simulink model "ofdm_txrx_mimo"  (Simulink 7.1)
settings: Solver=ode45  StartTime=0.0  StopTime=6e3  RelTol=1e-3
PreLoadFcn:
  | ofdm_rx_mimo_init
InitFcn:
  | ofdm_rx_mimo_init
StartFcn:
  | ofdm_rx_mimo_init
values from neighbouring files (symbols this model uses):
  BER_RAM_init_size = 2048    [ofdm_tx_mimo_init.m]
  CPLength = 16    [ofdm_tx_mimo_init.m]
  CRCPolynomial = hex2dec('04c11db7')    [ofdm_tx_mimo_init.m]
  freqOffset_track_filtCoef_i = hex2dec('2500')/2^32    [ofdm_rx_mimo_init.m]
  freqOffset_track_filtCoef_p = hex2dec('d0000')/2^32    [ofdm_rx_mimo_init.m]
  hi = zeros(1,length(cci))    [ofdm_rx_mimo_init.m]
  hr = zeros(1,length(ccr))    [ofdm_rx_mimo_init.m]
  max_CP_length = 16    [ofdm_tx_mimo_init.m]
  max_num_baseRateSymbols = 31    [ofdm_tx_mimo_init.m]
  max_num_subcarriers = 64    [ofdm_tx_mimo_init.m]
  max_num_trainingSymbols = 15    [ofdm_tx_mimo_init.m]
  max_numBytes = 16384    [ofdm_tx_mimo_init.m]
  max_OFDM_symbols = 2047    [ofdm_tx_mimo_init.m]
  modConstellation_qam16 = .75*[1 3 -1 -3]./3    [ofdm_tx_mimo_init.m]
  modConstellation_qam256 = 0.9375*[3 1 5 7 11 9 13 15 -3 -1 -5 -7 -11 -9 -13 -15]./15    [ofdm_tx_mimo_init.m]
  modConstellation_qam64 = 0.875*[3 1 5 7 -3 -1 -5 -7]./7    [ofdm_tx_mimo_init.m]
  modConstellation_qpsk = [1 -1]./sqrt(2)    [ofdm_tx_mimo_init.m]
  numBaseRateSymbols = 2    [ofdm_tx_mimo_init.m]
  numSubcarriers = 64    [ofdm_tx_mimo_init.m]
  numTrainingSymbols = 2    [ofdm_tx_mimo_init.m]
  pilot1_indicies = 7 + ( (64-7) * 2^16)    (= 3735559)    [ofdm_tx_mimo_init.m]
  pilot2_indicies = 21 + ( (64-21) * 2^16)    (= 2818069)    [ofdm_tx_mimo_init.m]
  pilotValue_neg = hex2dec('8000') + (2^16 * 0)    [ofdm_tx_mimo_init.m]
  pilotValue_pos = hex2dec('7FFF') + (2^16 * 0)    [ofdm_tx_mimo_init.m]
  pkt_crossCorr_thresh = 0.7    [ofdm_rx_mimo_init.m]
  pkt_energy_thresh = 0    [ofdm_rx_mimo_init.m]
  pkt_numPayloadBytes = 1400+42-6    (= 1436)    [ofdm_tx_mimo_init.m]
  pktByteNums = numHeaderBytes +  				byteIndex_numPayloadBytes(1)*2^8 +  				byteIndex_numPayloadBytes(2)*2^16 +  				byteIndex_simpleDynModMasks * 2^24    [ofdm_rx_mimo_init.m]
  PktDet_Delay = 26 + Symbol_Timing_Offset*2^7    (= 1050)    [ofdm_rx_mimo_init.m]
  preamble = 6*[0 shortsyms_10 longsyms_2]    [ofdm_tx_mimo_init.m]
  preamble_I = real(preamble)    [ofdm_tx_mimo_init.m]
  preamble_Q = imag(preamble)    [ofdm_tx_mimo_init.m]
  RAM_init_size = max_numBytes    (= 16384)    [ofdm_tx_mimo_init.m]
  reg_Interrupt_PktBuf_Control = reg_InterruptControl +  	1 * 2^16 +  	0 * 2^24    (= 65656)    [ofdm_rx_mimo_init.m]
  rx_controlBits = 1 * 2  		 + 1 * 4  		 + 0 * 8  		 + 1 * 16 * rx_SISO_Mode  		 + 1 * 32  		 + 1 * 64  		 + 0 * 128  		 + 1 * 256  		 + 0 * 512  		 + 1 * 1024  		 + 0 * 2048  		 + 1 * 4096  		 + 1 * 8192  		 + 0 * 16384  		 + 1 * 65536  		 + 1 * 131072  		 + 0 * 262144  		 + 1 * 524288    [ofdm_rx_mimo_init.m]
  Rx_PhaseNoiseTrack_K = 0.6094    [ofdm_rx_mimo_init.m]
  rx_postEq_scaling = round(rxScaling*2^11) * (1 + 2^16)    [ofdm_rx_mimo_init.m]
  RxDataScrambling_Seq = [40 198 78 63 82 173 102 245 48 111 172 115 147 230 216 93 72 65 62 2 205 242 122 90 128 83 105 97 73 10 5 252]    [ofdm_tx_mimo_init.m]
  simOnly_numSamples = length(preamble)+( (numSubcarriers+CPLength)*(numTrainingSymbols + numBaseRateSymbols + numFullRateSymbols/2) )    [ofdm_tx_mimo_init.m]
  train = [train train]    [ofdm_tx_mimo_init.m]
  tx_controlBits = (antB_preambleShift * 2^4) + (2^0 * tx_SISO_Mode) + 4*0 + 8*1    (= 73)    [ofdm_tx_mimo_init.m]
  TxDataScrambling_Seq = [40 198 78 63 82 173 102 245 48 111 172 115 147 230 216 93 72 65 62 2 205 242 122 90 128 83 105 97 73 10 5 252]    [ofdm_tx_mimo_init.m]
  txReg_symbolCounts = (2^16 * numFullRateSymbols) + (2^8 *numBaseRateSymbols ) + numTrainingSymbols    [ofdm_tx_mimo_init.m]
  TxRx_FFTScaling = bin2dec('010111') + (bin2dec('000101') * 2^6)    [ofdm_tx_mimo_init.m]
undefined symbols (not defined in the file or in its folder): AntA, AntB, BaseRate, CRC_table_gen, gcbh, intfilt, pktTiming_count_payloadStart, pktTiming_symbol_length, xlBlockLoadCallback, xlGetSimulinkPeriod
libraries: xbsIndex_r4 x4212, dspmlti4 x4, dspsigops x4, commchan3 x2, simulink x2, commrflib2 x2, dspsrcs4 x1
(part 9 of 43)

== system ofdm_txrx_mimo/OFDM Rx MIMO/Coarse Freq Correction/Coarse Freq Offset Calc Combo ==
  I In : Inport  OutDataType=sfix(16)  OutScaling=2^0
  Q In : Inport  Port=2  OutDataType=sfix(16)  OutScaling=2^0
  Reset : Inport  Port=3  OutDataType=sfix(16)  OutScaling=2^0
  PktDet : Inport  Port=4  OutDataType=sfix(16)  OutScaling=2^0
  Accum : xbsIndex_r4/Accumulator  operation=Add  n_bits=7+13  overflow=Flag as error  scale=1  rst=on  hasbypass=on  en=on  dbl_ovrd=off  use_behavioral_HDL=on  implementation=Fabric  block_type=accum
      settings: infoedit="Adder or subtractor-based accumulator.   Output type and binary point position match the input.<P><P>Hardware notes: When \"Reinitialize with input 'b' on reset\" is selected, the accumulator is forced to run at the system rate even if the input 'b' is running at a slower rate."  xl_area=[17 33 0 32 0 0 0]  block_version=VER_STRING_GOES_HERE
  Accum Control : Subsystem
  Arctan2 : Subsystem
  Coarse Freq Scope4 : Scope  NumInputPorts=5
      settings: TimeRange=3500  YMin=-5~-0.0001~0~0~0  YMax=12.5~0.00035~0.00035~1~1  SaveName=ScopeData43
  Convert1 : xbsIndex_r4/Convert  arith_type=Unsigned  n_bits=32  bin_pt=32  quantization=Truncate  overflow=Wrap  latency=0  dbl_ovrd=off  pipeline=off  block_type=convert
      settings: xl_area=[0 0 0 0 0 0 0]  block_version=VER_STRING_GOES_HERE
  Convert2 : xbsIndex_r4/Convert  arith_type="Signed  (2's comp)"  n_bits=14  bin_pt=11  quantization=Truncate  overflow=Wrap  latency=0  dbl_ovrd=off  pipeline=off  block_type=convert
      settings: xl_area=[0, 0, 0, 0, 0, 0, 0]  block_version=VER_STRING_GOES_HERE
  Convert3 : xbsIndex_r4/Convert  arith_type="Signed  (2's comp)"  n_bits=14  bin_pt=9  quantization=Truncate  overflow=Wrap  latency=0  dbl_ovrd=off  pipeline=off  block_type=convert
      settings: xl_area=[0, 0, 0, 0, 0, 0, 0]  block_version=VER_STRING_GOES_HERE
  Delay4 : xbsIndex_r4/Delay  en=off  latency=4  dbl_ovrd=off  reg_retiming=off  block_type=delay
      settings: infoedit=Hardware notes: A delay line is a chain, each link of which is an SRL16 followed by a flip-flop. If register retiming is enabled, the delay line is a chain of flip-flops.  block_version=VER_STRING_GOES_HERE
  Delay5 : xbsIndex_r4/Delay  en=off  latency=1  dbl_ovrd=off  reg_retiming=off  block_type=delay
      settings: infoedit=Hardware notes: A delay line is a chain, each link of which is an SRL16 followed by a flip-flop. If register retiming is enabled, the delay line is a chain of flip-flops.  block_version=VER_STRING_GOES_HERE
  Delay6 : xbsIndex_r4/Delay  en=off  latency=2  dbl_ovrd=off  reg_retiming=off  block_type=delay
      settings: infoedit=Hardware notes: A delay line is a chain, each link of which is an SRL16 followed by a flip-flop. If register retiming is enabled, the delay line is a chain of flip-flops.  block_version=VER_STRING_GOES_HERE
  Gateway Out{19..23} (5 alike) : xbsIndex_r4/Gateway Out  hdl_port=off  timing_constraint=None  locs_specified=off  LOCs={}  block_type=gatewayout
      settings: infoedit="Gateway out block.  Converts Xilinx fixed point inputs into ouputs of type Simulink integer, double, or fixed point.<P><P>Hardware notes:  In hardware these blocks become top level output ports or are discarded, depending on how they are configured."  xl_area=[0 0 0 0 0 0 0]  block_version=VER_STRING_GOES_HERE
  Inverter1 : xbsIndex_r4/Inverter  en=off  latency=0  dbl_ovrd=off  block_type=inv
      settings: xl_area=[0 0 0 0 0 0 0]  block_version=VER_STRING_GOES_HERE
  Logical : xbsIndex_r4/Logical  logical_function=AND  inputs=2  en=off  latency=0  precision=Full  arith_type=Unsigned  n_bits=16  bin_pt=0  align_bp=on  dbl_ovrd=off  block_type=logical
      settings: block_version=8.2.01
  Mult1 : xbsIndex_r4/Mult  precision=User Defined  arith_type="Signed  (2's comp)"  n_bits=35  bin_pt=33  quantization="Round  (unbiased: +/- Inf)"  overflow=Flag as error  en=off  latency=2  dbl_ovrd=off  use_behavioral_HDL=off  use_embedded=on  opt=Speed  optimum_pipeline=off  pipeline=off  use_rpm=off  placement_style=Rectangular shape  block_type=mult
      settings: infoedit=Hardware notes: To use the internal pipeline stage of the dedicated multiplier you must select 'Pipeline for maximum performance'.  xl_area=[52 69 0 102 0 2 0]  block_version=VER_STRING_GOES_HERE
  Mux : xbsIndex_r4/Mux  inputs=2  en=off  latency=1  precision=Full  arith_type=Unsigned  n_bits=16  bin_pt=14  quantization=Truncate  overflow=Wrap  dbl_ovrd=off  block_type=mux
      settings: block_version=8.2.01
  PhaseDiff : Subsystem
  Posedge : Subsystem
  Register4 : xbsIndex_r4/Register  init=0  rst=on  en=on  dbl_ovrd=off  block_type=register
      settings: block_version=8.2.01
  Rx_debug_CFOPhaseDiff : xbsIndex_r4/Gateway Out  hdl_port=on  timing_constraint=None  locs_specified=off  LOCs={}  block_type=gatewayout
      settings: infoedit="Gateway out block.  Converts Xilinx fixed point inputs into ouputs of type Simulink integer, double, or fixed point.<P><P>Hardware notes:  In hardware these blocks become top level output ports or are discarded, depending on how they are configured."  xl_area=[0 0 0 0 1 0 0]  block_version=VER_STRING_GOES_HERE
  Scale : xbsIndex_r4/Scale  scale_factor=-4  dbl_ovrd=off  block_type=scale
      settings: infoedit=Scales input by a power of two by adjusting the binary point position.<P><P>Hardware notes: In hardware this block costs nothing.  block_version=8.2.01
  Scale1 : xbsIndex_r4/Scale  scale_factor=-2  dbl_ovrd=off  block_type=scale
      settings: infoedit=Scales input by a power of two by adjusting the binary point position.<P><P>Hardware notes: In hardware this block costs nothing.  block_version=8.2.01
  Up Sample : xbsIndex_r4/Up Sample  sample_ratio=2  copy_samples=on  dbl_ovrd=off  block_type=usamp
      settings: infoedit="Up samples input data.  Inserted values  can be zeros or copies of the most recent input sample.<P><P>Hardware notes: No hardware is needed if inserted values are copies of the input sample; otherwise, a mux and single bit flip-flop are used."  block_version=8.2.02
  inv 2pi : xbsIndex_r4/Constant  arith_type=Unsigned  const=1/(2*pi*64*72)  n_bits=24  bin_pt=24  explicit_period=off  period=1  equ=P=C  opselect=C  inp2=PCIN>>17  opr=+  inp1=P  carry=CIN  dbl_ovrd=off  block_type=constant
      settings: block_version=VER_STRING_GOES_HERE
  Phase Inc : Outport  OutDataType=sfix(16)  OutScaling=2^0
  tag scope: CFO_PhaseDiff=global
  wires:
  PktDet/1 -> Accum Control/1(En)
  Q In/1 -> Arctan2/2(Q)
  I In/1 -> Arctan2/1(I)
  Accum Control/1(" En") -> Delay4/1
  Gateway Out19/1 -> Coarse Freq Scope4/1
  Gateway Out20/1 -> Coarse Freq Scope4/2
  Gateway Out21/1 -> Coarse Freq Scope4/3
  Gateway Out22/1 -> Coarse Freq Scope4/4
  Gateway Out23/1 -> Coarse Freq Scope4/5
  PhaseDiff/1(diff(p0,p63)) -> Accum/1, Convert3/1, [CFO_PhaseDiff]
  Inverter1/1 -> Accum/2, Posedge/1(D)
  Delay4/1 -> Delay5/1
  Accum/1 -> Mult1/1, Gateway Out22/1
  inv 2pi/1 -> Mult1/2
  Mult1/1 -> Register4/1, Gateway Out21/1
  Posedge/1(Q) -> Delay6/1
  Delay6/1 -> Gateway Out20/1, Logical/1
  Register4/1 -> Gateway Out19/1, Convert1/1
  Reset/1 -> Accum Control/2(Reset), Gateway Out23/1, Register4/2
  Arctan2/1(atan) -> PhaseDiff/1(p), Convert2/1
  Delay5/1 -> Inverter1/1, Accum/3
  Convert1/1 -> Phase Inc/1
  Logical/1 -> Register4/3
  [regRx_coarseCFO_en] -> Logical/2
  Up Sample/1 -> Rx_debug_CFOPhaseDiff/1
  Scale/1 -> Mux/3
  Mux/1 -> Up Sample/1
  [regRx_CFOdebugOutputSel] -> Mux/1
  Scale1/1 -> Mux/2
  Convert2/1 -> Scale1/1
  Convert3/1 -> Scale/1

== system ofdm_txrx_mimo/OFDM Rx MIMO/Coarse Freq Correction/DDS ==
  PhaseInc : Inport  OutDataType=sfix(16)  OutScaling=2^0
  Rst : Inport  Port=2  OutDataType=sfix(16)  OutScaling=2^0
  "DDS v4_0 " : xbsIndex_r4/DDS v4_0   Description=Direct Digital Synthesizer  mode=Sine and Cosine  neg_sin=on  neg_cos=off  n_bits=18  phase_increment_type=Register  phase_increment=1/10  phase_offset_type=Constant  phase_offset=0  rst=on  en=off  lut_addr_bits=10  acc_latency=One Cycle  acc_n_bits=32  phase_dithering=off  dbl_ovrd=off  explicit_period=off  period=1  mem_type=Block RAM  pipeline=off  use_rpm=off  block_type=ddsv4
      settings: block_version=VER_STRING_GOES_HERE
  Logical1 : xbsIndex_r4/Logical  logical_function=OR  inputs=2  en=off  latency=0  precision=Full  arith_type=Unsigned  n_bits=8  bin_pt=2  align_bp=on  dbl_ovrd=off  block_type=logical
      settings: xl_area=[1 0 0 1 0 0 0]  block_version=VER_STRING_GOES_HERE
  inv 2pi2 : xbsIndex_r4/Constant  arith_type=Boolean  const=1  n_bits=32  bin_pt=32  explicit_period=off  period=1  equ=P=C  opselect=C  inp2=PCIN>>17  opr=+  inp1=P  carry=CIN  dbl_ovrd=off  block_type=constant
      settings: block_version=VER_STRING_GOES_HERE
  Q : Outport  OutDataType=sfix(16)  OutScaling=2^0
  I : Outport  Port=2  OutDataType=sfix(16)  OutScaling=2^0
  wires:
  [regRx_freqCorrBypass] -> Logical1/2
  Logical1/1 -> "DDS v4_0 "/3
  PhaseInc/1 -> "DDS v4_0 "/1
  inv 2pi2/1 -> "DDS v4_0 "/2
  Rst/1 -> Logical1/1
  "DDS v4_0 "/1 -> Q/1
  "DDS v4_0 "/2 -> I/1

== system ofdm_txrx_mimo/OFDM Rx MIMO/Equalizer & Packetizer/CFO Loop Filter ==
  Phase Err : Inport  OutDataType=sfix(16)  OutScaling=2^0
  Rst : Inport  Port=2  OutDataType=sfix(16)  OutScaling=2^0
  Vin : Inport  Port=3  OutDataType=sfix(16)  OutScaling=2^0
  Accumulator : xbsIndex_r4/Accumulator  operation=Add  n_bits=32  overflow=Wrap  scale=1  rst=on  hasbypass=off  en=on  dbl_ovrd=off  use_behavioral_HDL=on  implementation=Fabric  block_type=accum
      settings: infoedit="Adder or subtractor-based accumulator.   Output type and binary point position match the input.<P><P>Hardware notes: When \"Reinitialize with input 'b' on reset\" is selected, the accumulator is forced to run at the system rate even if the input 'b' is running at a slower rate."  block_version=VER_STRING_GOES_HERE
  AddSub : xbsIndex_r4/AddSub  mode=Addition  use_carryin=off  use_carryout=off  en=off  latency=0  precision=User Defined  arith_type="Signed  (2's comp)"  n_bits=32  bin_pt=31  quantization=Truncate  overflow=Wrap  dbl_ovrd=off  use_behavioral_HDL=off  pipelined=off  use_rpm=on  hw_selection=Fabric  block_type=addsub
      settings: block_version=VER_STRING_GOES_HERE
  Delay : xbsIndex_r4/Delay  en=off  latency=3  dbl_ovrd=off  reg_retiming=off  block_type=delay
      settings: infoedit=Hardware notes: A delay line is a chain, each link of which is an SRL16 followed by a flip-flop. If register retiming is enabled, the delay line is a chain of flip-flops.  block_version=VER_STRING_GOES_HERE
  Display : Display
  Display1 : Display
  Down Sample1 : xbsIndex_r4/Down Sample  sample_ratio=2  sample_phase="Last Value of Frame  (most efficient)"  en=off  latency=1  dbl_ovrd=off  block_type=dsamp
      settings: block_version=VER_STRING_GOES_HERE
  Down Sample2 : xbsIndex_r4/Down Sample  sample_ratio=2  sample_phase="Last Value of Frame  (most efficient)"  en=off  latency=1  dbl_ovrd=off  block_type=dsamp
      settings: block_version=VER_STRING_GOES_HERE
  Gateway Out{1..6} (6 alike) : xbsIndex_r4/Gateway Out  hdl_port=off  timing_constraint=None  locs_specified=off  LOCs={}  block_type=gatewayout
      settings: infoedit="Gateway out block.  Converts Xilinx fixed point inputs into ouputs of type Simulink integer, double, or fixed point.<P><P>Hardware notes:  In hardware these blocks become top level output ports or are discarded, depending on how they are configured."  block_version=VER_STRING_GOES_HERE
  MIMO CFO Loop Filt : Scope  NumInputPorts=6
      settings: YMin=-3.5~-1.575e-006~-1.25e-005~-1.5e-005~0~-1  YMax=2.5~-1.375e-006~1e-005~1e-005~1~1  SaveName=ScopeData40
  Mult : xbsIndex_r4/Mult  precision=User Defined  arith_type="Signed  (2's comp)"  n_bits=32  bin_pt=31  quantization=Truncate  overflow=Wrap  en=off  latency=3  dbl_ovrd=off  use_behavioral_HDL=off  use_embedded=on  opt=Speed  optimum_pipeline=off  pipeline=on  use_rpm=off  placement_style=Rectangular shape  block_type=mult
      settings: infoedit=Hardware notes: To use the internal pipeline stage of the dedicated multiplier you must select 'Pipeline for maximum performance'.  block_version=VER_STRING_GOES_HERE
  Mult1 : xbsIndex_r4/Mult  precision=User Defined  arith_type="Signed  (2's comp)"  n_bits=32  bin_pt=31  quantization=Truncate  overflow=Wrap  en=off  latency=3  dbl_ovrd=off  use_behavioral_HDL=off  use_embedded=on  opt=Speed  optimum_pipeline=off  pipeline=on  use_rpm=off  placement_style=Rectangular shape  block_type=mult
      settings: infoedit=Hardware notes: To use the internal pipeline stage of the dedicated multiplier you must select 'Pipeline for maximum performance'.  block_version=VER_STRING_GOES_HERE
  Fine Freq : Outport  OutDataType=sfix(16)  OutScaling=2^0
  wires:
  [RxReg_FreqOffFilt_KI] -> Down Sample2/1
  Mult/1 -> Accumulator/1
  Accumulator/1 -> AddSub/1, Gateway Out1/1
  [RxReg_FreqOffFilt_KP] -> Down Sample1/1
  Phase Err/1 -> Mult1/2, Mult/1, Gateway Out2/1
  Mult1/1 -> AddSub/2, Gateway Out3/1
  AddSub/1 -> Gateway Out4/1, Fine Freq/1
  Gateway Out2/1 -> MIMO CFO Loop Filt/1
  Gateway Out1/1 -> MIMO CFO Loop Filt/2
  Gateway Out3/1 -> MIMO CFO Loop Filt/3
  Gateway Out4/1 -> MIMO CFO Loop Filt/4
  Rst/1 -> Accumulator/2, Gateway Out6/1
  Vin/1 -> Delay/1, Gateway Out5/1
  Delay/1 -> Accumulator/3
  Gateway Out5/1 -> MIMO CFO Loop Filt/5
  Gateway Out6/1 -> MIMO CFO Loop Filt/6
  Down Sample1/1 -> Mult1/1, Display/1
  Down Sample2/1 -> Mult/2, Display1/1

== system ofdm_txrx_mimo/OFDM Rx MIMO/Equalizer & Packetizer/EVM ==
  In1 : Inport  OutDataType=sfix(16)  OutScaling=2^0
  In2 : Inport  Port=2  OutDataType=sfix(16)  OutScaling=2^0
  In3 : Inport  Port=3  OutDataType=sfix(16)  OutScaling=2^0
  In4 : Inport  Orientation=down  Port=4  OutDataType=sfix(16)  OutScaling=2^0
  In5 : Inport  Orientation=down  Port=5  OutDataType=sfix(16)  OutScaling=2^0
  In6 : Inport  Orientation=down  Port=6  OutDataType=sfix(16)  OutScaling=2^0
  RST : Inport  Port=7  OutDataType=sfix(16)  OutScaling=2^0
  Accumulator : xbsIndex_r4/Accumulator  operation=Add  n_bits=32  overflow=Wrap  scale=1  rst=on  hasbypass=off  en=on  dbl_ovrd=off  use_behavioral_HDL=on  implementation=Fabric  block_type=accum
      settings: infoedit="Adder or subtractor-based accumulator.   Output type and binary point position match the input.<P><P>Hardware notes: When \"Reinitialize with input 'b' on reset\" is selected, the accumulator is forced to run at the system rate even if the input 'b' is running at a slower rate."  block_version=10.1.3
  AddSub : xbsIndex_r4/AddSub  mode=Subtraction  use_carryin=off  use_carryout=off  en=off  latency=0  precision=User Defined  arith_type="Signed  (2's comp)"  n_bits=18  bin_pt=15  quantization=Truncate  overflow=Wrap  dbl_ovrd=off  use_behavioral_HDL=off  pipelined=off  use_rpm=on  hw_selection=Fabric  block_type=addsub
      settings: block_version=10.1.3
  AddSub1 : xbsIndex_r4/AddSub  mode=Subtraction  use_carryin=off  use_carryout=off  en=off  latency=0  precision=User Defined  arith_type="Signed  (2's comp)"  n_bits=18  bin_pt=15  quantization=Truncate  overflow=Wrap  dbl_ovrd=off  use_behavioral_HDL=off  pipelined=off  use_rpm=on  hw_selection=Fabric  block_type=addsub
      settings: block_version=10.1.3
  AddSub2 : xbsIndex_r4/AddSub  mode=Addition  use_carryin=off  use_carryout=off  en=off  latency=0  precision=Full  arith_type=Unsigned  n_bits=16  bin_pt=14  quantization=Truncate  overflow=Wrap  dbl_ovrd=off  use_behavioral_HDL=off  pipelined=off  use_rpm=on  hw_selection=Fabric  block_type=addsub
      settings: block_version=10.1.3
  AddSub3 : xbsIndex_r4/AddSub  Orientation=down  mode=Subtraction  use_carryin=off  use_carryout=off  en=off  latency=0  precision=Full  arith_type=Unsigned  n_bits=16  bin_pt=14  quantization=Truncate  overflow=Wrap  dbl_ovrd=off  use_behavioral_HDL=off  pipelined=off  use_rpm=on  hw_selection=Fabric  block_type=addsub
      settings: block_version=10.1.3
  Bus Creator : BusCreator  Inputs=3
  Constant : xbsIndex_r4/Constant  arith_type=Unsigned  const=0  n_bits=4  bin_pt=0  explicit_period=off  period=1  equ=P=C  opselect=C  inp2=PCIN>>17  opr=+  inp1=P  carry=CIN  dbl_ovrd=off  block_type=constant
  Constant1 : xbsIndex_r4/Constant  Orientation=down  arith_type=Unsigned  const=1  n_bits=1  bin_pt=0  explicit_period=off  period=1  equ=P=C  opselect=C  inp2=PCIN>>17  opr=+  inp1=P  carry=CIN  dbl_ovrd=off  block_type=constant
  Constant2 : xbsIndex_r4/Constant  arith_type=Boolean  const=1  n_bits=1  bin_pt=0  explicit_period=off  period=1  equ=P=C  opselect=C  inp2=PCIN>>17  opr=+  inp1=P  carry=CIN  dbl_ovrd=off  block_type=constant
  Delay{,1..3,5} (5 alike) : xbsIndex_r4/Delay  Orientation=down  en=off  latency=2  dbl_ovrd=off  reg_retiming=off  block_type=delay
  Delay4 : xbsIndex_r4/Delay  en=off  latency=1  dbl_ovrd=off  reg_retiming=off  block_type=delay
  Delay6 : xbsIndex_r4/Delay  en=off  latency=1  dbl_ovrd=off  reg_retiming=off  block_type=delay
  Delay7 : xbsIndex_r4/Delay  Orientation=down  en=off  latency=6  dbl_ovrd=off  reg_retiming=off  block_type=delay
  Down Sample : xbsIndex_r4/Down Sample  Orientation=down  sample_ratio=80  sample_phase="Last Value of Frame  (most efficient)"  en=off  latency=1  dbl_ovrd=off  block_type=dsamp
      settings: block_version=10.1.3
  Inverter : xbsIndex_r4/Inverter  Orientation=down  en=off  latency=0  dbl_ovrd=off  block_type=inv
  Inverter1 : xbsIndex_r4/Inverter  en=off  latency=0  dbl_ovrd=off  block_type=inv
  Logical : xbsIndex_r4/Logical  logical_function=AND  inputs=2  en=off  latency=0  precision=Full  arith_type=Unsigned  n_bits=16  bin_pt=0  align_bp=on  dbl_ovrd=off  block_type=logical
  Logical1 : xbsIndex_r4/Logical  logical_function=AND  inputs=2  en=off  latency=0  precision=Full  arith_type=Unsigned  n_bits=16  bin_pt=0  align_bp=on  dbl_ovrd=off  block_type=logical
  Mult : xbsIndex_r4/Mult  precision=User Defined  arith_type="Signed  (2's comp)"  n_bits=16  bin_pt=11  quantization=Truncate  overflow=Wrap  en=off  latency=3  dbl_ovrd=off  use_behavioral_HDL=off  use_embedded=on  opt=Speed  optimum_pipeline=off  pipeline=on  use_rpm=on  placement_style=Triangular  block_type=mult
      settings: infoedit=Hardware notes: To use the internal pipeline stage of the dedicated multiplier you must select 'Pipeline for maximum performance'.  block_version=10.1.3
  Mult1 : xbsIndex_r4/Mult  precision=User Defined  arith_type="Signed  (2's comp)"  n_bits=16  bin_pt=11  quantization=Truncate  overflow=Wrap  en=off  latency=3  dbl_ovrd=off  use_behavioral_HDL=off  use_embedded=on  opt=Speed  optimum_pipeline=off  pipeline=on  use_rpm=on  placement_style=Triangular  block_type=mult
      settings: infoedit=Hardware notes: To use the internal pipeline stage of the dedicated multiplier you must select 'Pipeline for maximum performance'.  block_version=10.1.3
  Pkt Constructor Inputs1 : Scope  NumInputPorts=8
      settings: TimeRange=1800  YMin=-1.5~-1~-0.75~-0.75~0~-1~0~0  YMax=1.5~1~0.75~0.75~0.75~1~1~0.075  SaveName=ScopeData70
  Register : xbsIndex_r4/Register  init=0  rst=on  en=off  dbl_ovrd=off  block_type=register
  Register1 : xbsIndex_r4/Register  init=0  rst=off  en=on  dbl_ovrd=off  block_type=register
  Register2 : xbsIndex_r4/Register  init=0  rst=on  en=on  dbl_ovrd=off  block_type=register
  Relational : xbsIndex_r4/Relational  mode=a!=b  en=off  latency=1  dbl_ovrd=off  block_type=relational
      settings: block_version=10.1.3
  Relational1 : xbsIndex_r4/Relational  mode=a>b  en=off  latency=1  dbl_ovrd=off  block_type=relational
      settings: block_version=10.1.3
  To Register : xbsIndex_r4/To Register  shared_memory_name='Rx_collision'  init=0  ownership=Locally owned and initialized  explicit_data_type=on  arith_type=Unsigned  n_bits=1  bin_pt=0  dbl_ovrd=off  block_type=toreg
      settings: AttributesFormatString=<< %<shared_memory_name> >>  infoedit="Register block that writes data to a shared memory register.  Delay of one sample period."  block_version=10.1.3
  To Workspace : ToWorkspace  VariableName=simout  SampleTime=-1  SaveFormat=Structure
  done{2..5,11} (5 alike) : xbsIndex_r4/Gateway Out  hdl_port=off  timing_constraint=None  locs_specified=off  LOCs={}  block_type=gatewayout
      settings: infoedit="Gateway out block.  Converts Xilinx fixed point inputs into ouputs of type Simulink integer, double, or fixed point.<P><P>Hardware notes:  In hardware these blocks become top level output ports or are discarded, depending on how they are configured."  xl_area=[0 0 0 0 1 0 0]  block_version=VER_STRING_GOES_HERE
  wires:
  In1/1 -> done4/1, AddSub/2
  In2/1 -> done5/1, AddSub1/2
  AddSub/1 -> Mult/1, Mult/2
  AddSub1/1 -> Mult1/1, Mult1/2
  Mult/1 -> AddSub2/1
  Mult1/1 -> AddSub2/2
  AddSub2/1 -> Register/1
  Delay/1 -> AddSub/1, done2/1
  Delay1/1 -> AddSub1/1, done3/1
  Delay2/1 -> Delay4/1
  Constant/1 -> Relational/2
  Relational/1 -> Logical/1
  Logical/1 -> Delay3/1
  done2/1 -> Pkt Constructor Inputs1/1
  done3/1 -> Pkt Constructor Inputs1/2
  done4/1 -> Pkt Constructor Inputs1/3
  done5/1 -> Pkt Constructor Inputs1/4
  done11/1 -> Pkt Constructor Inputs1/5
  Register/1 -> Pkt Constructor Inputs1/8, Accumulator/1, Bus Creator/3
  Delay4/1 -> Logical/2, Delay5/1
  Delay3/1 -> Inverter/1, Accumulator/3
  Inverter/1 -> Register/2, Pkt Constructor Inputs1/7
  In4/1 -> Delay/1
  In5/1 -> Delay1/1
  In6/1 -> Delay2/1
  In3/1 -> Relational/1
  Delay5/1 -> Inverter1/1, Delay6/1
  Inverter1/1 -> Accumulator/2, Logical1/1
  Accumulator/1 -> Register1/1
  Register1/1 -> Down Sample/1, Bus Creator/2
  Delay6/1 -> Logical1/2
  Logical1/1 -> Register1/2
  Down Sample/1 -> AddSub3/1, Delay7/1
  Delay7/1 -> AddSub3/2
  AddSub3/1 -> done11/1, Bus Creator/1, Relational1/1
  Bus Creator/1 -> To Workspace/1
  [RxReg_EVM] -> Relational1/2
  Relational1/1 -> Register2/3
  Constant1/1 -> Register2/1
  RST/1 -> Register2/2
  Constant2/1 -> To Register/2
  Register2/1 -> To Register/1
  To Register/1 -> Pkt Constructor Inputs1/6

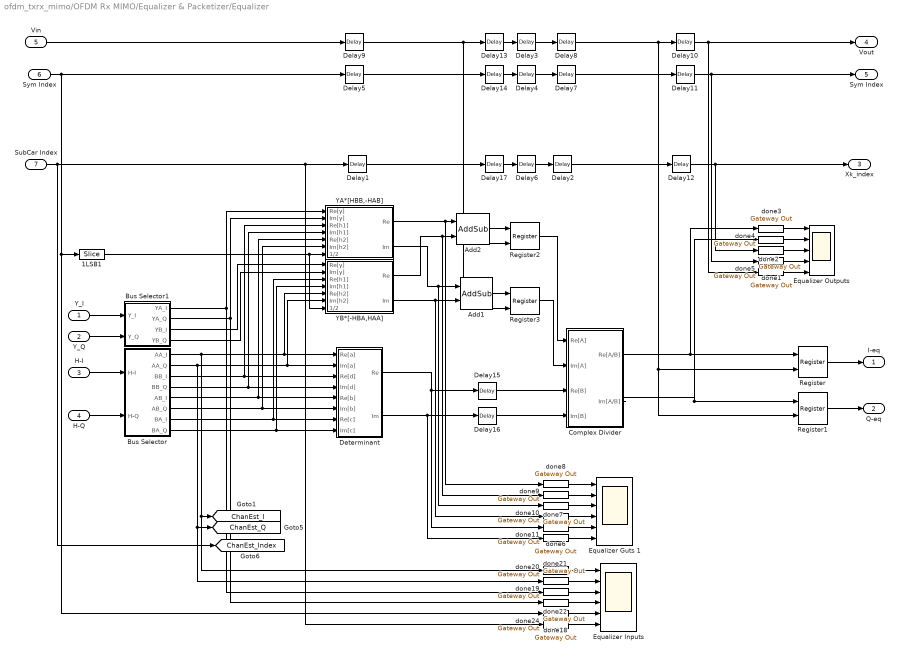
== system ofdm_txrx_mimo/OFDM Rx MIMO/Equalizer & Packetizer/Equalizer ==
  Y_I : Inport  OutDataType=sfix(16)  OutScaling=2^0
  Y_Q : Inport  Port=2  OutDataType=sfix(16)  OutScaling=2^0
  H-I : Inport  Port=3  OutDataType=sfix(16)  OutScaling=2^0
  H-Q : Inport  Port=4  OutDataType=sfix(16)  OutScaling=2^0
  Vin : Inport  Port=5  OutDataType=sfix(16)  OutScaling=2^0
  Sym Index : Inport  Port=6  OutDataType=sfix(16)  OutScaling=2^0
  SubCar Index : Inport  Port=7  OutDataType=sfix(16)  OutScaling=2^0
  1LSB1 : xbsIndex_r4/Slice  nbits=1  boolean_output=off  mode=Lower Bit Location + Width  bit1=0  base1=MSB of Input  bit0=0  base0=LSB of Input  dbl_ovrd=off  block_type=slice
      settings: infoedit="Extracts a given range of bits from each input sample and presents it at the output.  The output type is ordinarily unsigned with binary point at zero, but can be Boolean when the slice is one bit wide.<P><P>Hardware notes: In hardware this block costs nothing."  block_version=VER_STRING_GOES_HERE
  Add1 : xbsIndex_r4/AddSub  mode=Addition  use_carryin=off  use_carryout=off  en=off  latency=0  precision=Full  arith_type="Signed  (2's comp)"  n_bits=18  bin_pt=16  quantization=Truncate  overflow=Flag as error  dbl_ovrd=off  use_behavioral_HDL=on  pipelined=off  use_rpm=on  hw_selection=Fabric  block_type=addsub
      settings: xl_area=[9 0 0 16 0 0 0]  block_version=VER_STRING_GOES_HERE
  Add2 : xbsIndex_r4/AddSub  mode=Addition  use_carryin=off  use_carryout=off  en=off  latency=0  precision=Full  arith_type="Signed  (2's comp)"  n_bits=18  bin_pt=16  quantization=Truncate  overflow=Flag as error  dbl_ovrd=off  use_behavioral_HDL=on  pipelined=off  use_rpm=on  hw_selection=Fabric  block_type=addsub
      settings: xl_area=[9 0 0 16 0 0 0]  block_version=VER_STRING_GOES_HERE
  Bus Selector : Subsystem
  Bus Selector1 : Subsystem
  Complex Divider : Subsystem
  Delay{1,3..6,9} (6 alike) : xbsIndex_r4/Delay  en=off  latency=2  dbl_ovrd=off  reg_retiming=off  block_type=delay
      settings: infoedit=Hardware notes: A delay line is a chain, each link of which is an SRL16 followed by a flip-flop. If register retiming is enabled, the delay line is a chain of flip-flops.  xl_area=[0, 0, 0, 0, 0, 0, 0]  block_version=VER_STRING_GOES_HERE
  Delay{10..17} (8 alike) : xbsIndex_r4/Delay  en=off  latency=1  dbl_ovrd=off  reg_retiming=off  block_type=delay
      settings: infoedit=Hardware notes: A delay line is a chain, each link of which is an SRL16 followed by a flip-flop. If register retiming is enabled, the delay line is a chain of flip-flops.  xl_area=[0, 0, 0, 0, 0, 0, 0]  block_version=VER_STRING_GOES_HERE
  Delay2 : xbsIndex_r4/Delay  en=off  latency=19+2  dbl_ovrd=off  reg_retiming=off  block_type=delay
      settings: infoedit=Hardware notes: A delay line is a chain, each link of which is an SRL16 followed by a flip-flop. If register retiming is enabled, the delay line is a chain of flip-flops.  xl_area=[0, 0, 0, 0, 0, 0, 0]  block_version=VER_STRING_GOES_HERE
  Delay7 : xbsIndex_r4/Delay  en=off  latency=19+2  dbl_ovrd=off  reg_retiming=off  block_type=delay
      settings: infoedit=Hardware notes: A delay line is a chain, each link of which is an SRL16 followed by a flip-flop. If register retiming is enabled, the delay line is a chain of flip-flops.  xl_area=[0, 0, 0, 0, 0, 0, 0]  block_version=VER_STRING_GOES_HERE
  Delay8 : xbsIndex_r4/Delay  en=off  latency=19+2  dbl_ovrd=off  reg_retiming=off  block_type=delay
      settings: infoedit=Hardware notes: A delay line is a chain, each link of which is an SRL16 followed by a flip-flop. If register retiming is enabled, the delay line is a chain of flip-flops.  xl_area=[0, 0, 0, 0, 0, 0, 0]  block_version=VER_STRING_GOES_HERE
  Determinant : Subsystem
  Equalizer Outputs : Scope  NumInputPorts=5
      settings: TimeRange=4000  YMin=-0.0008~-0.0007~10~22.8~0.95  YMax=0.0007~0.0007~60~25.2~1.05  SaveName=ScopeData26
  Equalizer Guts 1 : Scope  NumInputPorts=6
      settings: TimeRange=5000  YMin=0~-0.001~-0.4~-0.2~0~0  YMax=0.8~0.001~0.4~0.8~1~80  SaveName=ScopeData58
  Equalizer Inputs : Scope  NumInputPorts=6
      settings: TimeRange=5000  YMin=0~-0.001~-0.4~-0.2~0~0  YMax=0.8~0.001~0.4~0.8~1~80  SaveName=ScopeData18
  Register{,1..3} (4 alike) : xbsIndex_r4/Register  init=0  rst=off  en=on  dbl_ovrd=off  block_type=register
      Register settings: block_version=VER_STRING_GOES_HERE
      Register1 settings: block_version=VER_STRING_GOES_HERE
      Register2 settings: block_version=8.2.02
      Register3 settings: block_version=8.2.02
  YA*[HBB,-HAB] : Subsystem
  YB*[-HBA,HAA] : Subsystem
  done{1..11,18..22,24} (17 alike) : xbsIndex_r4/Gateway Out  hdl_port=off  timing_constraint=None  locs_specified=off  LOCs={}  block_type=gatewayout
      settings: infoedit="Gateway out block.  Converts Xilinx fixed point inputs into ouputs of type Simulink integer, double, or fixed point.<P><P>Hardware notes:  In hardware these blocks become top level output ports or are discarded, depending on how they are configured."  xl_area=[0 0 0 0 1 0 0]  block_version=VER_STRING_GOES_HERE
  I-eq : Outport  OutDataType=sfix(16)  OutScaling=2^0
  Q-eq : Outport  Port=2  OutDataType=sfix(16)  OutScaling=2^0
  " Xk_index" : Outport  Port=3  OutDataType=sfix(16)  OutScaling=2^0
  Vout : Outport  Port=4  OutDataType=sfix(16)  OutScaling=2^0
  " Sym Index" : Outport  Port=5  OutDataType=sfix(16)  OutScaling=2^0
  tag scope: ChanEst_I=global, ChanEst_Index=global, ChanEst_Q=global
  wires:
  H-I/1 -> Bus Selector/1(H-I)
  H-Q/1 -> Bus Selector/2(H-Q)
  SubCar Index/1 -> [ChanEst_Index], Delay1/1, done24/1
  Delay6/1 -> Delay2/1
  done18/1 -> Equalizer Inputs/5
  done20/1 -> Equalizer Inputs/1
  done21/1 -> Equalizer Inputs/2
  done19/1 -> Equalizer Inputs/3
  done22/1 -> Equalizer Inputs/4
  done24/1 -> Equalizer Inputs/6
  done3/1 -> Equalizer Outputs/1
  done4/1 -> Equalizer Outputs/2
  done2/1 -> Equalizer Outputs/3
  Vin/1 -> Delay9/1
  Delay3/1 -> Delay8/1
  Delay4/1 -> Delay7/1
  done1/1 -> Equalizer Outputs/4
  Delay2/1 -> Delay12/1
  Delay7/1 -> Delay11/1
  Delay8/1 -> Register1/2, Register/2, Delay10/1
  Sym Index/1 -> Delay5/1, 1LSB1/1, done18/1
  Bus Selector/1(AA_I) -> Determinant/1(Re[a]), YB*[-HBA,HAA]/5(Re[h2]), done20/1, [ChanEst_I]
  Bus Selector/2(AA_Q) -> Determinant/2(Im[a]), YB*[-HBA,HAA]/6(Im[h2]), done21/1, [ChanEst_Q]
  Bus Selector/3(BB_I) -> Determinant/3(Re[d]), YA*[HBB,-HAB]/3(Re[h1])
  Bus Selector/4(BB_Q) -> Determinant/4(Im[d]), YA*[HBB,-HAB]/4(Im[h1])
  Bus Selector/5(AB_I) -> Determinant/5(Re[b]), YA*[HBB,-HAB]/5(Re[h2])
  Bus Selector/6(AB_Q) -> Determinant/6(Im[b]), YA*[HBB,-HAB]/6(Im[h2])
  Bus Selector/7(BA_I) -> Determinant/7(Re[c]), YB*[-HBA,HAA]/3(Re[h1])
  Bus Selector/8(BA_Q) -> Determinant/8(Im[c]), YB*[-HBA,HAA]/4(Im[h1])
  Determinant/1(Re) -> done6/1, Delay15/1
  Determinant/2(Im) -> done11/1, Delay16/1
  Y_I/1 -> Bus Selector1/1(Y_I)
  Y_Q/1 -> Bus Selector1/2(Y_Q)
  Bus Selector1/1(YA_I) -> YA*[HBB,-HAB]/1(Re[y]), done19/1
  Bus Selector1/2(YA_Q) -> YA*[HBB,-HAB]/2(Im[y]), done22/1
  Bus Selector1/3(YB_I) -> YB*[-HBA,HAA]/1(Re[y])
  Bus Selector1/4(YB_Q) -> YB*[-HBA,HAA]/2(Im[y])
  YA*[HBB,-HAB]/1(Re) -> done8/1, Add2/1
  YB*[-HBA,HAA]/1(Re) -> done9/1, Add2/2
  YA*[HBB,-HAB]/2(Im) -> done7/1, Add1/1
  YB*[-HBA,HAA]/2(Im) -> done10/1, Add1/2
  Delay1/1 -> Delay17/1
  Delay5/1 -> Delay14/1
  Delay9/1 -> Delay13/1, Register2/2, Register3/2
  done5/1 -> Equalizer Outputs/5
  Complex Divider/1(Re[A/B]) -> Register/1, done3/1
  Register/1 -> I-eq/1
  Complex Divider/2(Im[A/B]) -> Register1/1, done4/1
  Register1/1 -> Q-eq/1
  Delay10/1 -> Vout/1, done5/1
  Delay11/1 -> " Sym Index"/1, done1/1
  Delay12/1 -> " Xk_index"/1, done2/1
  done6/1 -> Equalizer Guts 1/5
  done8/1 -> Equalizer Guts 1/1
  done9/1 -> Equalizer Guts 1/2
  done7/1 -> Equalizer Guts 1/3
  done10/1 -> Equalizer Guts 1/4
  done11/1 -> Equalizer Guts 1/6
  1LSB1/1 -> YA*[HBB,-HAB]/7(1/2), YB*[-HBA,HAA]/7(1/2)
  Add2/1 -> Register2/1
  Add1/1 -> Register3/1
  Delay14/1 -> Delay4/1
  Delay13/1 -> Delay3/1
  Register2/1 -> Complex Divider/1(Re[A])
  Register3/1 -> Complex Divider/2(Im[A])
  Delay15/1 -> Complex Divider/3(Re[B])
  Delay16/1 -> Complex Divider/4(Im[B])
  Delay17/1 -> Delay6/1

== system ofdm_txrx_mimo/OFDM Rx MIMO/Equalizer & Packetizer/Packet_Constructor ==
  note: The index keeps track of the first unused location in the buffer, indexed from zero.
      |   I.e. if there are 5 bits in the buffer occupying 0 to 4, the index will be 5.
      | This is used to select which byte out of the 15 bits in the buffer will make it into the RAM block.
  I : Inport  OutDataType=sfix(16)  OutScaling=2^0
  Q : Inport  Port=2  OutDataType=sfix(16)  OutScaling=2^0
  Xk_index : Inport  Port=3  OutDataType=sfix(16)  OutScaling=2^0
  Vin : Inport  Port=4  OutDataType=sfix(16)  OutScaling=2^0
  Sym Index : Inport  Port=5  OutDataType=sfix(16)  OutScaling=2^0
  Start : Inport  Port=6  OutDataType=sfix(16)  OutScaling=2^0
  "  " : Subsystem  mask{period=1}
  >=8? : xbsIndex_r4/Slice  Orientation=up  nbits=1  boolean_output=off  mode=Lower Bit Location + Width  bit1=0  base1=MSB of Input  bit0=3  base0=LSB of Input  dbl_ovrd=off  block_type=slice
      settings: infoedit="Extracts a given range of bits from each input sample and presents it at the output.  The output type is ordinarily unsigned with binary point at zero, but can be Boolean when the slice is one bit wide.<P><P>Hardware notes: In hardware this block costs nothing."  block_version=VER_STRING_GOES_HERE
  AddSub1 : xbsIndex_r4/AddSub  mode=Subtraction  use_carryin=off  use_carryout=off  en=off  latency=0  precision=User Defined  arith_type=Unsigned  n_bits=4  bin_pt=0  quantization=Truncate  overflow=Wrap  dbl_ovrd=off  use_behavioral_HDL=on  pipelined=off  use_rpm=on  hw_selection=Fabric  block_type=addsub
      settings: xl_area=[2 0 0 4 0 0 0]  block_version=VER_STRING_GOES_HERE
  AddSub3 : xbsIndex_r4/AddSub  mode=Addition  use_carryin=off  use_carryout=off  en=off  latency=0  precision=User Defined  arith_type=Unsigned  n_bits=4  bin_pt=0  quantization=Truncate  overflow=Wrap  dbl_ovrd=off  use_behavioral_HDL=on  pipelined=off  use_rpm=on  hw_selection=Fabric  block_type=addsub
      settings: xl_area=[2 0 0 4 0 0 0]  block_version=VER_STRING_GOES_HERE
  Assert1 : xbsIndex_r4/Assert  Orientation=left  assert_type=off  type_source=Explicitly  arith_type="Signed  (2's comp)"  n_bits=16  bin_pt=14  assert_rate=on  rate_source=Explicitly  period=2  output_port=on  block_type=assert
      settings: infoedit=Asserts a user-defined sample rate and/or type on a signal.<P><P>Hardware notes: In hardware this block costs nothing.  block_version=VER_STRING_GOES_HERE
  Assert2 : xbsIndex_r4/Assert  assert_type=off  type_source=Explicitly  arith_type="Signed  (2's comp)"  n_bits=16  bin_pt=14  assert_rate=on  rate_source=Explicitly  period=2  output_port=on  block_type=assert
      settings: infoedit=Asserts a user-defined sample rate and/or type on a signal.<P><P>Hardware notes: In hardware this block costs nothing.  block_version=VER_STRING_GOES_HERE
  Barrel Shifter : Subsystem
  Convert2 : xbsIndex_r4/Convert  Orientation=down  arith_type=Boolean  n_bits=8  bin_pt=6  quantization=Truncate  overflow=Wrap  latency=0  dbl_ovrd=off  pipeline=off  block_type=convert
      settings: xl_area=[0 0 0 0 0 0 0]  block_version=VER_STRING_GOES_HERE
  Convert3 : xbsIndex_r4/Convert  Orientation=down  arith_type=Unsigned  n_bits=3  bin_pt=0  quantization=Truncate  overflow=Wrap  latency=0  dbl_ovrd=off  pipeline=off  block_type=convert
      settings: xl_area=[0 0 0 0 0 0 0]  block_version=VER_STRING_GOES_HERE
  Counter1 : xbsIndex_r4/Counter  cnt_type=Free Running  cnt_to=Inf  operation=Up  start_count=0  cnt_by_val=1  arith_type=Unsigned  n_bits=ceil(log2(RAM_init_size))  bin_pt=0  load_pin=off  rst=on  en=on  explicit_period=off  period=2  dbl_ovrd=off  use_behavioral_HDL=off  use_rpm=on  implementation=Fabric  block_type=counter
      settings: xl_area=[5 10 0 10 0 0 0]  block_version=VER_STRING_GOES_HERE
  Data Buffer : Subsystem
  Delay1 : xbsIndex_r4/Delay  en=off  latency=1  dbl_ovrd=off  reg_retiming=off  block_type=delay
      settings: infoedit=Hardware notes: A delay line is a chain, each link of which is an SRL16 followed by a flip-flop. If register retiming is enabled, the delay line is a chain of flip-flops.  xl_area=[1 1 0 0 0 0 0]  block_version=VER_STRING_GOES_HERE
  Delay2 : xbsIndex_r4/Delay  en=off  latency=1  dbl_ovrd=off  reg_retiming=off  block_type=delay
      settings: infoedit=Hardware notes: A delay line is a chain, each link of which is an SRL16 followed by a flip-flop. If register retiming is enabled, the delay line is a chain of flip-flops.  xl_area=[1 1 0 0 0 0 0]  block_version=VER_STRING_GOES_HERE
  Delay3 : xbsIndex_r4/Delay  en=off  latency=1  dbl_ovrd=off  reg_retiming=off  block_type=delay
      settings: infoedit=Hardware notes: A delay line is a chain, each link of which is an SRL16 followed by a flip-flop. If register retiming is enabled, the delay line is a chain of flip-flops.  xl_area=[1 1 0 0 0 0 0]  block_version=VER_STRING_GOES_HERE
  Hard Decision : Subsystem
  Inverter : xbsIndex_r4/Inverter  en=off  latency=0  dbl_ovrd=off  block_type=inv
      settings: xl_area=[0 0 0 0 0 0 0]  block_version=VER_STRING_GOES_HERE
  Inverter1 : xbsIndex_r4/Inverter  en=off  latency=0  dbl_ovrd=off  block_type=inv
      settings: xl_area=[0 0 0 0 0 0 0]  block_version=VER_STRING_GOES_HERE
  Logical1 : xbsIndex_r4/Logical  Orientation=down  logical_function=AND  inputs=3  en=off  latency=0  precision=Full  arith_type=Unsigned  n_bits=8  bin_pt=2  align_bp=on  dbl_ovrd=off  block_type=logical
      settings: xl_area=[1 0 0 1 0 0 0]  block_version=VER_STRING_GOES_HERE
  Logical2 : xbsIndex_r4/Logical  logical_function=AND  inputs=3  en=off  latency=0  precision=Full  arith_type=Unsigned  n_bits=8  bin_pt=2  align_bp=on  dbl_ovrd=off  block_type=logical
      settings: xl_area=[1 0 0 1 0 0 0]  block_version=VER_STRING_GOES_HERE
  Logical3 : xbsIndex_r4/Logical  logical_function=AND  inputs=2  en=off  latency=0  precision=Full  arith_type=Unsigned  n_bits=8  bin_pt=2  align_bp=on  dbl_ovrd=off  block_type=logical
      settings: xl_area=[1 0 0 1 0 0 0]  block_version=VER_STRING_GOES_HERE
  Logical4 : xbsIndex_r4/Logical  logical_function=OR  inputs=3  en=off  latency=0  precision=Full  arith_type=Unsigned  n_bits=8  bin_pt=2  align_bp=on  dbl_ovrd=off  block_type=logical
      settings: xl_area=[4 0 0 4 0 0 0]  block_version=VER_STRING_GOES_HERE
  Modulation RAM : Subsystem
  Pkt Interrupt Outputs : Subsystem
  Register : xbsIndex_r4/Register  init=0  rst=off  en=off  dbl_ovrd=off  block_type=register
      settings: block_version=8.2.02
  Relational : xbsIndex_r4/Relational  mode=a<b  en=off  latency=0  dbl_ovrd=off  block_type=relational
      settings: xl_area=[6 0 0 11 0 0 0]  block_version=VER_STRING_GOES_HERE
  Shift8 : xbsIndex_r4/Shift  Orientation=left  shift_dir=Left  shift_bits=3  en=off  latency=0  precision=User Defined  arith_type=Unsigned  n_bits=4  bin_pt=0  quantization=Truncate  overflow=Wrap  dbl_ovrd=off  block_type=shift
      settings: xl_area=[0 0 0 0 0 0 0]  block_version=VER_STRING_GOES_HERE
  Slicer : Subsystem
  index acc1 : xbsIndex_r4/Accumulator  operation=Add  n_bits=4  overflow=Wrap  scale=1  rst=on  hasbypass=off  en=on  dbl_ovrd=off  use_behavioral_HDL=on  implementation=Fabric  block_type=accum
      settings: infoedit="Adder or subtractor-based accumulator.   Output type and binary point position match the input.<P><P>Hardware notes: When \"Reinitialize with input 'b' on reset\" is selected, the accumulator is forced to run at the system rate even if the input 'b' is running at a slower rate."  xl_area=[2 4 0 4 0 0 0]  block_version=VER_STRING_GOES_HERE
  done : Outport  OutDataType=sfix(16)  OutScaling=2^0
  IOUT : Outport  Port=2  OutDataType=sfix(16)  OutScaling=2^0
  QOUT : Outport  Port=3  OutDataType=sfix(16)  OutScaling=2^0
  MOD : Outport  Port=4  OutDataType=sfix(16)  OutScaling=2^0
  tag scope: EQ_I=global, EQ_Q=global
  wires:
  Delay2/1 -> AddSub1/1, AddSub3/1, Barrel Shifter/1(ShiftSel)
  Data Buffer/1(numBytes) -> Relational/2
  Logical4/1 -> index acc1/2, Counter1/1, Data Buffer/4(Rst)
  AddSub1/1 -> index acc1/1
  Shift8/1 -> AddSub1/2
  index acc1/1 -> AddSub3/2
  AddSub3/1 -> Assert2/1
  >=8?/1 -> Shift8/1, Convert2/1
  Barrel Shifter/1(Q) -> Slicer/2(D)
  Logical2/1 -> Data Buffer/2(Payload_WE)
  Slicer/1(Q) -> Data Buffer/3(Payload_Data)
  "  "/1(pulse) -> Register/1
  Inverter/1 -> "  "/1(signal)
  Convert2/1 -> Logical1/2, Logical2/2
  Logical1/1 -> Counter1/2
  Logical3/1 -> Logical2/1, Logical1/3, Inverter/1
  Counter1/1 -> Data Buffer/1(Payload_Addr), Relational/1
  Convert3/1 -> Slicer/1(SliceSel)
  Modulation RAM/1(mod) -> Hard Decision/1(Mod Sel), Delay2/1
  Xk_index/1 -> Modulation RAM/1(Xk_index)
  Sym Index/1 -> Modulation RAM/2(Sym Index)
  Hard Decision/1(bits) -> Barrel Shifter/2(D)
  I/1 -> Hard Decision/2(I), [EQ_I]
  Q/1 -> Hard Decision/3(Q), [EQ_Q]
  Start/1 -> Logical4/1
  [rx_global_reset] -> Logical4/2, Logical4/3
  Data Buffer/2(CRCError) -> Pkt Interrupt Outputs/2(CRC-Err)
  Assert2/1 -> Convert3/1, >=8?/1
  Vin/1 -> Delay3/1
  Delay1/1 -> Barrel Shifter/3(en), index acc1/3, Logical1/1, Logical2/3
  Delay3/1 -> Delay1/1
  Register/1 -> done/1, Pkt Interrupt Outputs/1(PktDone)
  Data Buffer/6(modMask) -> Modulation RAM/3(FullRateMasks)
  Data Buffer/5(ResetRx) -> Assert1/1
  Relational/1 -> Logical3/1
  Inverter1/1 -> Logical3/2
  Assert1/1 -> Inverter1/1
  Data Buffer/3(GoodHeader) -> Pkt Interrupt Outputs/3(GoodHeader)
  Data Buffer/4(BadHeader) -> Pkt Interrupt Outputs/4(BadHeader)
  Hard Decision/2(IOUT) -> IOUT/1
  Hard Decision/3(QOUT) -> QOUT/1
  Hard Decision/4(MOD) -> MOD/1

== system ofdm_txrx_mimo/OFDM Rx MIMO/Equalizer & Packetizer/Phase Adjust ==
  Phase : Inport  OutDataType=sfix(16)  OutScaling=2^0
  I : Inport  Port=2  OutDataType=sfix(16)  OutScaling=2^0
  Q : Inport  Port=3  OutDataType=sfix(16)  OutScaling=2^0
  SubCar Index : Inport  Port=4  OutDataType=sfix(16)  OutScaling=2^0
  Vin : Inport  Port=5  OutDataType=sfix(16)  OutScaling=2^0
  Sym Index : Inport  Port=6  OutDataType=sfix(16)  OutScaling=2^0
  ComplexMult_x1 : Subsystem
  Delay{1..4} (4 alike) : xbsIndex_r4/Delay  en=off  latency=1  dbl_ovrd=off  reg_retiming=off  block_type=delay
      settings: infoedit=Hardware notes: A delay line is a chain, each link of which is an SRL16 followed by a flip-flop. If register retiming is enabled, the delay line is a chain of flip-flops.  xl_area=[0, 0, 0, 0, 0, 0, 0]  block_version=VER_STRING_GOES_HERE
  Delay5 : xbsIndex_r4/Delay  en=off  latency=4  dbl_ovrd=off  reg_retiming=off  block_type=delay
      settings: infoedit=Hardware notes: A delay line is a chain, each link of which is an SRL16 followed by a flip-flop. If register retiming is enabled, the delay line is a chain of flip-flops.  xl_area=[0, 0, 0, 0, 0, 0, 0]  block_version=VER_STRING_GOES_HERE
  Delay6 : xbsIndex_r4/Delay  en=off  latency=4  dbl_ovrd=off  reg_retiming=off  block_type=delay
      settings: infoedit=Hardware notes: A delay line is a chain, each link of which is an SRL16 followed by a flip-flop. If register retiming is enabled, the delay line is a chain of flip-flops.  xl_area=[0, 0, 0, 0, 0, 0, 0]  block_version=VER_STRING_GOES_HERE
  Delay7 : xbsIndex_r4/Delay  en=off  latency=4  dbl_ovrd=off  reg_retiming=off  block_type=delay
      settings: infoedit=Hardware notes: A delay line is a chain, each link of which is an SRL16 followed by a flip-flop. If register retiming is enabled, the delay line is a chain of flip-flops.  xl_area=[0, 0, 0, 0, 0, 0, 0]  block_version=VER_STRING_GOES_HERE
  Phase Adjust : Scope  NumInputPorts=7
      settings: TimeRange=1800  YMin=-0.75~0.425~-1~-1.25~-1.25~-1.25~-1  YMax=0.75~0.65~1~1.25~1.25~1.25~1  SaveName=ScopeData67
  Phase Calc : Subsystem
  SineCosine : xbsIndex_r4/SineCosine  mode=Sine and Cosine  neg_sin=on  neg_cos=off  n_bits=16  sym_output=off  en=off  latency=1  dbl_ovrd=off  explicit_period=off  period=1  mem_type=Block RAM  pipeline=off  use_rpm=on  block_type=sincos
      settings: block_version=8.2.02
  done{1..2,6..10} (7 alike) : xbsIndex_r4/Gateway Out  hdl_port=off  timing_constraint=None  locs_specified=off  LOCs={}  block_type=gatewayout
      settings: infoedit="Gateway out block.  Converts Xilinx fixed point inputs into ouputs of type Simulink integer, double, or fixed point.<P><P>Hardware notes:  In hardware these blocks become top level output ports or are discarded, depending on how they are configured."  xl_area=[0 0 0 0 1 0 0]  block_version=VER_STRING_GOES_HERE
  " I" : Outport  OutDataType=sfix(16)  OutScaling=2^0
  " Q" : Outport  Port=2  OutDataType=sfix(16)  OutScaling=2^0
  " SubCar Index" : Outport  Port=3  OutDataType=sfix(16)  OutScaling=2^0
  Vout : Outport  Port=4  OutDataType=sfix(16)  OutScaling=2^0
  " Sym Index" : Outport  Port=5  OutDataType=sfix(16)  OutScaling=2^0
  wires:
  SineCosine/2 -> done7/1, ComplexMult_x1/3(Re[B])
  Vin/1 -> Delay5/1
  Sym Index/1 -> Delay6/1
  SubCar Index/1 -> Delay7/1
  Delay7/1 -> " SubCar Index"/1
  Delay6/1 -> " Sym Index"/1
  Delay5/1 -> Vout/1
  Delay1/1 -> ComplexMult_x1/1(Re[A]), done8/1
  Delay2/1 -> ComplexMult_x1/2(Im[A]), done9/1
  SineCosine/1 -> ComplexMult_x1/4(Im[B]), done1/1
  done6/1 -> Phase Adjust/1
  done7/1 -> Phase Adjust/2
  done1/1 -> Phase Adjust/3
  done8/1 -> Phase Adjust/4
  done9/1 -> Phase Adjust/5
  done10/1 -> Phase Adjust/6
  done2/1 -> Phase Adjust/7
  ComplexMult_x1/1(Re) -> done10/1, " I"/1
  ComplexMult_x1/2(Im) -> done2/1, " Q"/1
  I/1 -> Delay4/1
  Q/1 -> Delay3/1
  Phase Calc/1(" Phase") -> SineCosine/1, done6/1
  Phase/1 -> Phase Calc/1(Phase/k)
  Delay3/1 -> Delay2/1
  Delay4/1 -> Delay1/1

== system ofdm_txrx_mimo/OFDM Rx MIMO/Equalizer & Packetizer/Phase Noise Tracker ==
  note: http://ieeexplore.ieee.org/xpls/abs_all.jsp?arnumber=1683187
  note: Adapted from the phase tracking system described by Akita, Sakata and Sato
  note: http://sciencelinks.jp/j-east/article/200415/000020041504A0494348.php
  note: http://ieeexplore.ieee.org/xpls/abs_all.jsp?arnumber=1405003
  Phase Err : Inport  OutDataType=sfix(16)  OutScaling=2^0
  Reset : Inport  Port=2  OutDataType=sfix(16)  OutScaling=2^0
  Vin : Inport  Port=3  OutDataType=sfix(16)  OutScaling=2^0
  Sym Index : Inport  Port=4  OutDataType=sfix(16)  OutScaling=2^0
  Coarse Tracking : Subsystem
  Fine Tracking : Subsystem
  theta : Outport  OutDataType=sfix(16)  OutScaling=2^0
  wires:
  Phase Err/1 -> Coarse Tracking/1(Phase Err)
  Reset/1 -> Coarse Tracking/2(Rst), Fine Tracking/4(Rst)
  Vin/1 -> Coarse Tracking/3(Vin)
  Coarse Tracking/1(phi) -> Fine Tracking/1(phi)
  Coarse Tracking/2(theta_coarse) -> Fine Tracking/2(theta_coarse)
  Sym Index/1 -> Fine Tracking/5(Sym Index)
  Coarse Tracking/3(vout) -> Fine Tracking/3(Vin)
  Fine Tracking/1(theta) -> theta/1
